simulink model "MX_Gateway"  (Simulink 9.0)
settings: Solver=FixedStepDiscrete  StartTime=0.0  StopTime=100  FixedStep=0.01  RelTol=1e-3
values from neighbouring files (symbols this model uses):
  ACTIVE = Bobcat.Parameter; CreateDefine( ACTIVE                         ,1                          ,'Define' ,'defines.h' )    [defines.m]
  ACTIVE_MODE_ONE = Bobcat.Parameter; CreateDefine( ACTIVE_MODE_ONE                ,19                         ,'Define' ,'defines.h' )    [defines.m]
  AUTHENTICATED = Bobcat.Parameter; CreateDefine( AUTHENTICATED                  ,1                          ,'Define' ,'defines.h' )    [defines.m]
  AUX_EMEA_CONTROLS = Bobcat.Parameter; CreateDefine( AUX_EMEA_CONTROLS  ,1   ,'Define' ,'defines.h' )    [enums.m]
  AUX_FLOW_HIGH = Bobcat.Parameter; CreateDefine( AUX_FLOW_HIGH           ,4  ,'Define' ,'defines.h' )    [enums.m]
  AUX_FLOW_INACTIVE = Bobcat.Parameter; CreateDefine( AUX_FLOW_INACTIVE       ,0  ,'Define' ,'defines.h' )    [enums.m]
  AUX_FLOW_LOW = Bobcat.Parameter; CreateDefine( AUX_FLOW_LOW            ,1  ,'Define' ,'defines.h' )    [enums.m]
  AUX_FLOW_MEDIUM = Bobcat.Parameter; CreateDefine( AUX_FLOW_MEDIUM         ,2  ,'Define' ,'defines.h' )    [enums.m]
  AUX_FLOW_STANDARD = Bobcat.Parameter; CreateDefine( AUX_FLOW_STANDARD       ,3  ,'Define' ,'defines.h' )    [enums.m]
  AUX_NON_FUNCTIONAL = Bobcat.Parameter; CreateDefine( AUX_NON_FUNCTIONAL ,255 ,'Define' ,'defines.h' )    [enums.m]
  BASE_ACTIVE = Bobcat.Parameter; CreateDefine( BASE_ACTIVE                    ,253                        ,'Define' ,'defines.h' )    [defines.m]
  BASE_DETENT = Bobcat.Parameter; CreateDefine( BASE_DETENT                    ,1                          ,'Define' ,'defines.h' )    [defines.m]
  BEEP_NONE = Bobcat.Parameter; CreateDefine( BEEP_NONE                      ,255                        ,'Define' ,'defines.h' )    [defines.m]
  CD_AIR_FILTER_DEBOUNCE_NORMAL = Bobcat.Parameter; CreateAliasCD( CD_AIR_FILTER_DEBOUNCE_NORMAL                        ,uint16(500)                ,'ConstantDataPointer' ,'CONSTANT_DATA' ,'AIR_FILTER_DEBOUNCE_NORMAL')    [cdata.m]    (also in hc12_cdata.m; m4_cdata.m)
  CD_AIR_FILTER_DEBOUNCE_NOT_CONNECTED = Bobcat.Parameter; CreateAliasCD( CD_AIR_FILTER_DEBOUNCE_NOT_CONNECTED                 ,uint16(3000)               ,'ConstantDataPointer' ,'CONSTANT_DATA' ,'AIR_FILTER_DEBOUNCE_NOT_CONNECTED')    [cdata.m]    (also in hc12_cdata.m; m4_cdata.m)
  CD_AIR_FILTER_DEBOUNCE_PLUGGED = Bobcat.Parameter; CreateAliasCD( CD_AIR_FILTER_DEBOUNCE_PLUGGED                       ,uint16(3000)               ,'ConstantDataPointer' ,'CONSTANT_DATA' ,'AIR_FILTER_DEBOUNCE_PLUGGED')    [cdata.m]    (also in hc12_cdata.m; m4_cdata.m)
  CD_AIR_FILTER_FUNCTION = Bobcat.Parameter; CreateAliasCD( CD_AIR_FILTER_FUNCTION                               ,uint8(255)                 ,'ConstantDataPointer' ,'CONSTANT_DATA' ,'AIR_FILTER_FUNCTION')    [cdata.m]    (also in hc12_cdata.m; m4_cdata.m)
  CD_AIR_FILTER_PLUGGED_WEIGHT = Bobcat.Parameter; CreateAliasCD( CD_AIR_FILTER_PLUGGED_WEIGHT                         ,uint8(2)                   ,'ConstantDataPointer' ,'CONSTANT_DATA' ,'AIR_FILTER_PLUGGED_WEIGHT')    [cdata.m]    (also in hc12_cdata.m; m4_cdata.m)
  CD_AIR_FILTER_UNPLUGGED_WEIGHT = Bobcat.Parameter; CreateAliasCD( CD_AIR_FILTER_UNPLUGGED_WEIGHT                       ,uint8(1)                   ,'ConstantDataPointer' ,'CONSTANT_DATA' ,'AIR_FILTER_UNPLUGGED_WEIGHT')    [cdata.m]    (also in hc12_cdata.m; m4_cdata.m)
  CD_AUTOIDLE_ARMBAR_DELAY = Bobcat.Parameter; CreateAliasCD( CD_AUTOIDLE_ARMBAR_DELAY                             ,uint8(2)                   ,'ConstantDataPointer' ,'CONSTANT_DATA' ,'AUTOIDLE_ARMBAR_DELAY')    [cdata.m]    (also in hc12_cdata.m; m4_cdata.m)
  CD_AUTOIDLE_FUNCTION = Bobcat.Parameter; CreateAliasCD( CD_AUTOIDLE_FUNCTION                                 ,uint8(255)                 ,'ConstantDataPointer' ,'CONSTANT_DATA' ,'AUTOIDLE_FUNCTION')    [cdata.m]    (also in hc12_cdata.m; m4_cdata.m)
  CD_AUTOIDLE_IDLE_DELAY = Bobcat.Parameter; CreateAliasCD( CD_AUTOIDLE_IDLE_DELAY                               ,uint8(40)                  ,'ConstantDataPointer' ,'CONSTANT_DATA' ,'AUTOIDLE_IDLE_DELAY')    [cdata.m]    (also in hc12_cdata.m; m4_cdata.m)
  CD_AUTOIDLE_LOW_IDLE_START = Bobcat.Parameter; CreateAliasCD( CD_AUTOIDLE_LOW_IDLE_START                           ,uint8(255)                 ,'ConstantDataPointer' ,'CONSTANT_DATA' ,'AUTOIDLE_LOW_IDLE_START')    [cdata.m]    (also in hc12_cdata.m; m4_cdata.m)
  CD_AUTOIDLE_PRESSURE_OFF = Bobcat.Parameter; CreateAliasCD( CD_AUTOIDLE_PRESSURE_OFF                             ,uint16(300)                ,'ConstantDataPointer' ,'CONSTANT_DATA' ,'AUTOIDLE_PRESSURE_OFF')    [cdata.m]    (also in hc12_cdata.m; m4_cdata.m)
  CD_AUTOIDLE_PRESSURE_ON = Bobcat.Parameter; CreateAliasCD( CD_AUTOIDLE_PRESSURE_ON                              ,uint16(270)                ,'ConstantDataPointer' ,'CONSTANT_DATA' ,'AUTOIDLE_PRESSURE_ON')    [cdata.m]    (also in hc12_cdata.m; m4_cdata.m)
  CD_AUTOSHIFT_FUNCTION = Bobcat.Parameter; CreateAliasCD( CD_AUTOSHIFT_FUNCTION                                ,uint8(255)                 ,'ConstantDataPointer' ,'CONSTANT_DATA' ,'AUTOSHIFT_FUNCTION')    [cdata.m]    (also in hc12_cdata.m; m4_cdata.m)
  CD_AUTOSHIFT_PHIGH1 = Bobcat.Parameter; CreateAliasCD( CD_AUTOSHIFT_PHIGH1                                  ,uint16(205)                ,'ConstantDataPointer' ,'CONSTANT_DATA' ,'AUTOSHIFT_PHIGH1')    [cdata.m]    (also in hc12_cdata.m; m4_cdata.m)
  CD_AUTOSHIFT_PHIGH2 = Bobcat.Parameter; CreateAliasCD( CD_AUTOSHIFT_PHIGH2                                  ,uint16(193)                ,'ConstantDataPointer' ,'CONSTANT_DATA' ,'AUTOSHIFT_PHIGH2')    [cdata.m]    (also in hc12_cdata.m; m4_cdata.m)
  CD_AUTOSHIFT_PHIGH_TEMP1 = Bobcat.Parameter; CreateAliasCD( CD_AUTOSHIFT_PHIGH_TEMP1                             ,int16(70)                  ,'ConstantDataPointer' ,'CONSTANT_DATA' ,'AUTOSHIFT_PHIGH_TEMP1')    [cdata.m]    (also in hc12_cdata.m; m4_cdata.m)
  CD_AUTOSHIFT_PHIGH_TEMP2 = Bobcat.Parameter; CreateAliasCD( CD_AUTOSHIFT_PHIGH_TEMP2                             ,int16(160)                 ,'ConstantDataPointer' ,'CONSTANT_DATA' ,'AUTOSHIFT_PHIGH_TEMP2')    [cdata.m]    (also in hc12_cdata.m; m4_cdata.m)
  CD_AUTOSHIFT_PLOW1 = Bobcat.Parameter; CreateAliasCD( CD_AUTOSHIFT_PLOW1                                   ,uint16(130)                ,'ConstantDataPointer' ,'CONSTANT_DATA' ,'AUTOSHIFT_PLOW1')    [cdata.m]    (also in hc12_cdata.m; m4_cdata.m)
  CD_AUTOSHIFT_PLOW2 = Bobcat.Parameter; CreateAliasCD( CD_AUTOSHIFT_PLOW2                                   ,uint16(100)                ,'ConstantDataPointer' ,'CONSTANT_DATA' ,'AUTOSHIFT_PLOW2')    [cdata.m]    (also in hc12_cdata.m; m4_cdata.m)
  CD_AUTOSHIFT_PLOW_TEMP1 = Bobcat.Parameter; CreateAliasCD( CD_AUTOSHIFT_PLOW_TEMP1                              ,int16(20)                  ,'ConstantDataPointer' ,'CONSTANT_DATA' ,'AUTOSHIFT_PLOW_TEMP1')    [cdata.m]    (also in hc12_cdata.m; m4_cdata.m)
  CD_AUTOSHIFT_PLOW_TEMP2 = Bobcat.Parameter; CreateAliasCD( CD_AUTOSHIFT_PLOW_TEMP2                              ,int16(80)                  ,'ConstantDataPointer' ,'CONSTANT_DATA' ,'AUTOSHIFT_PLOW_TEMP2')    [cdata.m]    (also in hc12_cdata.m; m4_cdata.m)
  CD_AUTOSHIFT_TRAVEL_DELAY = Bobcat.Parameter; CreateAliasCD( CD_AUTOSHIFT_TRAVEL_DELAY                            ,uint8(32)                  ,'ConstantDataPointer' ,'CONSTANT_DATA' ,'AUTOSHIFT_TRAVEL_DELAY')    [cdata.m]    (also in hc12_cdata.m; m4_cdata.m)
  CD_AUX2_CRACKPOINT_CURRENT = Bobcat.Parameter; CreateAliasCD( CD_AUX2_CRACKPOINT_CURRENT                           ,fi(0,0,16,12,'hex','0c28') ,'ConstantDataPointer' ,'CONSTANT_DATA' ,'AUX2_CRACKPOINT_CURRENT')    [cdata.m]    (also in hc12_cdata.m; m4_cdata.m)
  CD_AUX2_FLOW_LOW_FUNCTION = Bobcat.Parameter; CreateAliasCD( CD_AUX2_FLOW_LOW_FUNCTION                            ,uint8(255)                 ,'ConstantDataPointer' ,'CONSTANT_DATA' ,'AUX2_FLOW_LOW_FUNCTION')    [cdata.m]    (also in hc12_cdata.m; m4_cdata.m)
  CD_AUX2_FLOW_MED_FUNCTION = Bobcat.Parameter; CreateAliasCD( CD_AUX2_FLOW_MED_FUNCTION                            ,uint8(255)                 ,'ConstantDataPointer' ,'CONSTANT_DATA' ,'AUX2_FLOW_MED_FUNCTION')    [cdata.m]    (also in hc12_cdata.m; m4_cdata.m)
  CD_AUX2_HI_FLOW_PERCENT = Bobcat.Parameter; CreateAliasCD( CD_AUX2_HI_FLOW_PERCENT                              ,fi(0,0,16,16,'hex','ffff') ,'ConstantDataPointer' ,'CONSTANT_DATA' ,'AUX2_HI_FLOW_PERCENT')    [cdata.m]    (also in hc12_cdata.m; m4_cdata.m)
  CD_AUX2_LOW_FLOW_PERCENT = Bobcat.Parameter; CreateAliasCD( CD_AUX2_LOW_FLOW_PERCENT                             ,fi(0,0,16,16,'hex','7333') ,'ConstantDataPointer' ,'CONSTANT_DATA' ,'AUX2_LOW_FLOW_PERCENT')    [cdata.m]    (also in hc12_cdata.m; m4_cdata.m)
  CD_AUX2_MAX_FLOW_CURRENT = Bobcat.Parameter; CreateAliasCD( CD_AUX2_MAX_FLOW_CURRENT                             ,fi(0,0,16,12,'hex','14cc') ,'ConstantDataPointer' ,'CONSTANT_DATA' ,'AUX2_MAX_FLOW_CURRENT')    [cdata.m]    (also in hc12_cdata.m; m4_cdata.m)
  CD_AUX2_MED_FLOW_PERCENT = Bobcat.Parameter; CreateAliasCD( CD_AUX2_MED_FLOW_PERCENT                             ,fi(0,0,16,16,'hex','9999') ,'ConstantDataPointer' ,'CONSTANT_DATA' ,'AUX2_MED_FLOW_PERCENT')    [cdata.m]    (also in hc12_cdata.m; m4_cdata.m)
  CD_AUX_CRACKPOINT_CURRENT = Bobcat.Parameter; CreateAliasCD( CD_AUX_CRACKPOINT_CURRENT                            ,fi(0,0,16,12,'hex','0000') ,'ConstantDataPointer' ,'CONSTANT_DATA' ,'AUX_CRACKPOINT_CURRENT')    [cdata.m]    (also in hc12_cdata.m; m4_cdata.m)
  CD_AUX_DETENT_FLASH_TIME = Bobcat.Parameter; CreateAliasCD( CD_AUX_DETENT_FLASH_TIME                             ,uint16(72)                 ,'ConstantDataPointer' ,'CONSTANT_DATA' ,'AUX_DETENT_FLASH_TIME')    [cdata.m]    (also in hc12_cdata.m; m4_cdata.m)
  CD_AUX_DETENT_HOLD_TIME = Bobcat.Parameter; CreateAliasCD( CD_AUX_DETENT_HOLD_TIME                              ,uint16(100)                ,'ConstantDataPointer' ,'CONSTANT_DATA' ,'AUX_DETENT_HOLD_TIME')    [cdata.m]    (also in hc12_cdata.m; m4_cdata.m)
  CD_AUX_FULL_ON_AUX1 = Bobcat.Parameter; CreateAliasCD( CD_AUX_FULL_ON_AUX1                                  ,fi(0,0,16,12,'hex','10cd') ,'ConstantDataPointer' ,'CONSTANT_DATA' ,'AUX_FULL_ON_AUX1')    [cdata.m]    (also in hc12_cdata.m; m4_cdata.m)
  CD_AUX_FULL_ON_AUX2 = Bobcat.Parameter; CreateAliasCD( CD_AUX_FULL_ON_AUX2                                  ,fi(0,0,16,12,'hex','139d') ,'ConstantDataPointer' ,'CONSTANT_DATA' ,'AUX_FULL_ON_AUX2')    [cdata.m]    (also in hc12_cdata.m; m4_cdata.m)
  CD_AUX_FULL_ON_AUX3 = Bobcat.Parameter; CreateAliasCD( CD_AUX_FULL_ON_AUX3                                  ,fi(0,0,16,12,'hex','17d7') ,'ConstantDataPointer' ,'CONSTANT_DATA' ,'AUX_FULL_ON_AUX3')    [cdata.m]    (also in hc12_cdata.m; m4_cdata.m)
  CD_AUX_FUNCTION = Bobcat.Parameter; CreateAliasCD( CD_AUX_FUNCTION                                      ,uint8(0)                   ,'ConstantDataPointer' ,'CONSTANT_DATA' ,'AUX_FUNCTION')    [cdata.m]    (also in hc12_cdata.m; m4_cdata.m)
  CD_AUX_RATE_LIMIT_CRACKPOINT = Bobcat.Parameter; CreateAliasCD( CD_AUX_RATE_LIMIT_CRACKPOINT                         ,fi(0,0,16,12,'hex','0800') ,'ConstantDataPointer' ,'CONSTANT_DATA' ,'AUX_RATE_LIMIT_CRACKPOINT')    [cdata.m]    (also in hc12_cdata.m; m4_cdata.m)
  CD_AUX_RATE_LIMIT_CRACKPOINT_RATE = Bobcat.Parameter; CreateAliasCD( CD_AUX_RATE_LIMIT_CRACKPOINT_RATE                    ,fi(0,1,32,12,'int',102400) ,'ConstantDataPointer' ,'CONSTANT_DATA' ,'AUX_RATE_LIMIT_CRACKPOINT_RATE')    [cdata.m]    (also in hc12_cdata.m; m4_cdata.m)
  CD_AUX_RATE_LIMIT_FUNCTION = Bobcat.Parameter; CreateAliasCD( CD_AUX_RATE_LIMIT_FUNCTION                           ,uint8(255)                 ,'ConstantDataPointer' ,'CONSTANT_DATA' ,'AUX_RATE_LIMIT_FUNCTION')    [cdata.m]    (also in hc12_cdata.m; m4_cdata.m)
  CD_AUX_RATE_LIMIT_MAX_ENG_SPD = Bobcat.Parameter; CreateAliasCD( CD_AUX_RATE_LIMIT_MAX_ENG_SPD                        ,uint16(1600)               ,'ConstantDataPointer' ,'CONSTANT_DATA' ,'AUX_RATE_LIMIT_MAX_ENG_SPD')    [cdata.m]    (also in hc12_cdata.m; m4_cdata.m)
  CD_AUX_RATE_LIMIT_MIN_ENG_SPD = Bobcat.Parameter; CreateAliasCD( CD_AUX_RATE_LIMIT_MIN_ENG_SPD                        ,uint16(100)                ,'ConstantDataPointer' ,'CONSTANT_DATA' ,'AUX_RATE_LIMIT_MIN_ENG_SPD')    [cdata.m]    (also in hc12_cdata.m; m4_cdata.m)
  CD_AUX_RATE_LIMIT_NORMAL_RATE = Bobcat.Parameter; CreateAliasCD( CD_AUX_RATE_LIMIT_NORMAL_RATE                        ,fi(0,1,32,12,'int',2048)   ,'ConstantDataPointer' ,'CONSTANT_DATA' ,'AUX_RATE_LIMIT_NORMAL_RATE')    [cdata.m]    (also in hc12_cdata.m; m4_cdata.m)
  CD_AUXPOT_CAL_DEADBAND_HIGH = Bobcat.Parameter; CreateAliasCD( CD_AUXPOT_CAL_DEADBAND_HIGH                          ,uint16(574)                ,'ConstantDataPointer' ,'CONSTANT_DATA' ,'AUXPOT_CAL_DEADBAND_HIGH')    [cdata.m]    (also in hc12_cdata.m; m4_cdata.m)
  CD_AUXPOT_CAL_DEADBAND_LOW = Bobcat.Parameter; CreateAliasCD( CD_AUXPOT_CAL_DEADBAND_LOW                           ,uint16(424)                ,'ConstantDataPointer' ,'CONSTANT_DATA' ,'AUXPOT_CAL_DEADBAND_LOW')    [cdata.m]    (also in hc12_cdata.m; m4_cdata.m)
  CD_AUXPOT_CENTER_RATIO = Bobcat.Parameter; CreateAliasCD( CD_AUXPOT_CENTER_RATIO                               ,uint16(500)                ,'ConstantDataPointer' ,'CONSTANT_DATA' ,'AUXPOT_CENTER_RATIO')    [cdata.m]    (also in hc12_cdata.m; m4_cdata.m)
  CD_AUXPOT_DEADBAND = Bobcat.Parameter; CreateAliasCD( CD_AUXPOT_DEADBAND                                   ,uint8(38)                  ,'ConstantDataPointer' ,'CONSTANT_DATA' ,'AUXPOT_DEADBAND')    [cdata.m]    (also in hc12_cdata.m; m4_cdata.m)
  CD_AUXPOT_FUNCTION = Bobcat.Parameter; CreateAliasCD( CD_AUXPOT_FUNCTION                                   ,uint8(0)                   ,'ConstantDataPointer' ,'CONSTANT_DATA' ,'AUXPOT_FUNCTION')    [cdata.m]    (also in hc12_cdata.m; m4_cdata.m)
  CD_AUXPOT_RATIO_GAIN = Bobcat.Parameter; CreateAliasCD( CD_AUXPOT_RATIO_GAIN                                 ,uint16(1000)               ,'ConstantDataPointer' ,'CONSTANT_DATA' ,'AUXPOT_RATIO_GAIN')    [cdata.m]    (also in hc12_cdata.m; m4_cdata.m)
  CD_CAN_THROTTLE_FUNCTION = Bobcat.Parameter; CreateAliasCD( CD_CAN_THROTTLE_FUNCTION                             ,uint8(255)                 ,'ConstantDataPointer' ,'CONSTANT_DATA' ,'CAN_THROTTLE_FUNCTION')    [cdata.m]    (also in hc12_cdata.m; m4_cdata.m)
  CD_CAN_THROTTLE_REVERSE = Bobcat.Parameter; CreateAliasCD( CD_CAN_THROTTLE_REVERSE                              ,uint8(255)                 ,'ConstantDataPointer' ,'CONSTANT_DATA' ,'CAN_THROTTLE_REVERSE')    [cdata.m]    (also in hc12_cdata.m; m4_cdata.m)
  CD_CONSOLE_DEBOUNCE_ABSENT = Bobcat.Parameter; CreateAliasCD( CD_CONSOLE_DEBOUNCE_ABSENT                           ,uint16(12)                 ,'ConstantDataPointer' ,'CONSTANT_DATA' ,'CONSOLE_DEBOUNCE_ABSENT')    [cdata.m]    (also in hc12_cdata.m; m4_cdata.m)
  CD_CONSOLE_DEBOUNCE_OORH = Bobcat.Parameter; CreateAliasCD( CD_CONSOLE_DEBOUNCE_OORH                             ,uint16(12)                 ,'ConstantDataPointer' ,'CONSTANT_DATA' ,'CONSOLE_DEBOUNCE_OORH')    [cdata.m]    (also in hc12_cdata.m; m4_cdata.m)
  CD_CONSOLE_DEBOUNCE_OORL = Bobcat.Parameter; CreateAliasCD( CD_CONSOLE_DEBOUNCE_OORL                             ,uint16(12)                 ,'ConstantDataPointer' ,'CONSTANT_DATA' ,'CONSOLE_DEBOUNCE_OORL')    [cdata.m]    (also in hc12_cdata.m; m4_cdata.m)
  CD_CONSOLE_DEBOUNCE_PRESENT = Bobcat.Parameter; CreateAliasCD( CD_CONSOLE_DEBOUNCE_PRESENT                          ,uint16(12)                 ,'ConstantDataPointer' ,'CONSTANT_DATA' ,'CONSOLE_DEBOUNCE_PRESENT')    [cdata.m]    (also in hc12_cdata.m; m4_cdata.m)
  CD_CONSOLE_FUNCTION = Bobcat.Parameter; CreateAliasCD( CD_CONSOLE_FUNCTION                                  ,uint8(0)                   ,'ConstantDataPointer' ,'CONSTANT_DATA' ,'CONSOLE_FUNCTION')    [cdata.m]    (also in hc12_cdata.m; m4_cdata.m)
  CD_CONSOLE_OORH = Bobcat.Parameter; CreateAliasCD( CD_CONSOLE_OORH                                      ,uint16(740)                ,'ConstantDataPointer' ,'CONSTANT_DATA' ,'CONSOLE_OORH')    [cdata.m]    (also in hc12_cdata.m; m4_cdata.m)
  CD_CONSOLE_OORL = Bobcat.Parameter; CreateAliasCD( CD_CONSOLE_OORL                                      ,uint16(10)                 ,'ConstantDataPointer' ,'CONSTANT_DATA' ,'CONSOLE_OORL')    [cdata.m]    (also in hc12_cdata.m; m4_cdata.m)
  CD_CONSOLE_PRESENT_HIGH = Bobcat.Parameter; CreateAliasCD( CD_CONSOLE_PRESENT_HIGH                              ,uint16(515)                ,'ConstantDataPointer' ,'CONSTANT_DATA' ,'CONSOLE_PRESENT_HIGH')    [cdata.m]    (also in hc12_cdata.m; m4_cdata.m)
  CD_CONSOLE_PRESENT_LOW = Bobcat.Parameter; CreateAliasCD( CD_CONSOLE_PRESENT_LOW                               ,uint16(375)                ,'ConstantDataPointer' ,'CONSTANT_DATA' ,'CONSOLE_PRESENT_LOW')    [cdata.m]    (also in hc12_cdata.m; m4_cdata.m)
  CD_DELUXE_GEN5_5_FUNCTION = Bobcat.Parameter; CreateAliasCD( CD_DELUXE_GEN5_5_FUNCTION                            ,uint8(255)                 ,'ConstantDataPointer' ,'CONSTANT_DATA' ,'DELUXE_GEN5_5_FUNCTION')    [cdata.m]    (also in hc12_cdata.m; m4_cdata.m)
  CD_DELUXE_GEN5_FUNCTION = Bobcat.Parameter; CreateAliasCD( CD_DELUXE_GEN5_FUNCTION                              ,uint8(255)                 ,'ConstantDataPointer' ,'CONSTANT_DATA' ,'DELUXE_GEN5_FUNCTION')    [cdata.m]    (also in hc12_cdata.m; m4_cdata.m)
  CD_DERATE_ENGINE_SPEED_DEBOUNCE_HIGH = Bobcat.Parameter; CreateAliasCD( CD_DERATE_ENGINE_SPEED_DEBOUNCE_HIGH                 ,uint16(200)                ,'ConstantDataPointer' ,'CONSTANT_DATA' ,'DERATE_ENGINE_SPEED_DEBOUNCE_HIGH')    [cdata.m]    (also in hc12_cdata.m; m4_cdata.m)
  CD_DERATE_ENGINE_SPEED_DEBOUNCE_SHUTDOWN = Bobcat.Parameter; CreateAliasCD( CD_DERATE_ENGINE_SPEED_DEBOUNCE_SHUTDOWN             ,uint16(1000)               ,'ConstantDataPointer' ,'CONSTANT_DATA' ,'DERATE_ENGINE_SPEED_DEBOUNCE_SHUTDOWN')    [cdata.m]    (also in hc12_cdata.m; m4_cdata.m)
  CD_DERATE_ENGINE_SPEED_HIGH_OFFSET = Bobcat.Parameter; CreateAliasCD( CD_DERATE_ENGINE_SPEED_HIGH_OFFSET                   ,uint16(200)                ,'ConstantDataPointer' ,'CONSTANT_DATA' ,'DERATE_ENGINE_SPEED_HIGH_OFFSET')    [cdata.m]    (also in hc12_cdata.m; m4_cdata.m)
  CD_DERATE_METHOD = Bobcat.Parameter; CreateAliasCD( CD_DERATE_METHOD                                     ,uint8(0)                   ,'ConstantDataPointer' ,'CONSTANT_DATA' ,'DERATE_METHOD')    [cdata.m]    (also in hc12_cdata.m; m4_cdata.m)
  CD_DUMP_VALVE_FUNCTION = Bobcat.Parameter; CreateAliasCD( CD_DUMP_VALVE_FUNCTION                               ,uint8(255)                 ,'ConstantDataPointer' ,'CONSTANT_DATA' ,'DUMP_VALVE_FUNCTION')    [cdata.m]    (also in hc12_cdata.m; m4_cdata.m)
  CD_DUMP_VALVE_ON_CURRENT = Bobcat.Parameter; CreateAliasCD( CD_DUMP_VALVE_ON_CURRENT                             ,fi(0,0,16,12,'hex','ffff') ,'ConstantDataPointer' ,'CONSTANT_DATA' ,'DUMP_VALVE_ON_CURRENT')    [cdata.m]    (also in hc12_cdata.m; m4_cdata.m)
  CD_ECO_MODE_THROTTLE_REDUCTION = Bobcat.Parameter; CreateAliasCD( CD_ECO_MODE_THROTTLE_REDUCTION                       ,uint16(850)                ,'ConstantDataPointer' ,'CONSTANT_DATA' ,'ECO_MODE_THROTTLE_REDUCTION')    [cdata.m]    (also in hc12_cdata.m; m4_cdata.m)
  CD_ECT_FILTER_GAIN = Bobcat.Parameter; CreateAliasCD( CD_ECT_FILTER_GAIN                                   ,uint8(6)                   ,'ConstantDataPointer' ,'CONSTANT_DATA' ,'ECT_FILTER_GAIN')    [cdata.m]    (also in hc12_cdata.m; m4_cdata.m)
  CD_ECU_FUNCTION = Bobcat.Parameter; CreateAliasCD( CD_ECU_FUNCTION                                      ,uint8(255)                 ,'ConstantDataPointer' ,'CONSTANT_DATA' ,'ECU_FUNCTION')    [cdata.m]    (also in hc12_cdata.m; m4_cdata.m)
  CD_ECU_HOLD_DEBOUNCE_ERROR_ON = Bobcat.Parameter; CreateAliasCD( CD_ECU_HOLD_DEBOUNCE_ERROR_ON                        ,uint16(20)                 ,'ConstantDataPointer' ,'CONSTANT_DATA' ,'ECU_HOLD_DEBOUNCE_ERROR_ON')    [cdata.m]    (also in hc12_cdata.m; m4_cdata.m)
  CD_EMEA_CONTROLS_HOLD_TIME = Bobcat.Parameter; CreateAliasCD( CD_EMEA_CONTROLS_HOLD_TIME                           ,uint16(30)                 ,'ConstantDataPointer' ,'CONSTANT_DATA' ,'EMEA_CONTROLS_HOLD_TIME')    [cdata.m]    (also in hc12_cdata.m; m4_cdata.m)
  CD_EMEA_CONTROLS_OPTION_A = Bobcat.Parameter; CreateAliasCD( CD_EMEA_CONTROLS_OPTION_A                            ,uint8(255)                 ,'ConstantDataPointer' ,'CONSTANT_DATA' ,'EMEA_CONTROLS_OPTION_A')    [cdata.m]    (also in hc12_cdata.m; m4_cdata.m)
  CD_EMEA_CONTROLS_OPTION_C = Bobcat.Parameter; CreateAliasCD( CD_EMEA_CONTROLS_OPTION_C                            ,uint8(255)                 ,'ConstantDataPointer' ,'CONSTANT_DATA' ,'EMEA_CONTROLS_OPTION_C')    [cdata.m]    (also in hc12_cdata.m; m4_cdata.m)
  CD_EMEA_CONTROLS_REVERSE = Bobcat.Parameter; CreateAliasCD( CD_EMEA_CONTROLS_REVERSE                             ,uint8(255)                 ,'ConstantDataPointer' ,'CONSTANT_DATA' ,'EMEA_CONTROLS_REVERSE')    [cdata.m]    (also in hc12_cdata.m; m4_cdata.m)
  CD_EMEA_ELECTRIC_VERSION = Bobcat.Parameter; CreateAliasCD( CD_EMEA_ELECTRIC_VERSION                             ,uint8(0)                   ,'ConstantDataPointer' ,'CONSTANT_DATA' ,'EMEA_ELECTRIC_VERSION')    [cdata.m]    (also in hc12_cdata.m; m4_cdata.m)
  CD_EMEA_POT_CAL_DEADBAND_HIGH = Bobcat.Parameter; CreateAliasCD( CD_EMEA_POT_CAL_DEADBAND_HIGH                        ,uint16(574)                ,'ConstantDataPointer' ,'CONSTANT_DATA' ,'EMEA_POT_CAL_DEADBAND_HIGH')    [cdata.m]    (also in hc12_cdata.m; m4_cdata.m)
  CD_EMEA_POT_CAL_DEADBAND_LOW = Bobcat.Parameter; CreateAliasCD( CD_EMEA_POT_CAL_DEADBAND_LOW                         ,uint16(424)                ,'ConstantDataPointer' ,'CONSTANT_DATA' ,'EMEA_POT_CAL_DEADBAND_LOW')    [cdata.m]    (also in hc12_cdata.m; m4_cdata.m)
  CD_ENGINE_RPM_FACTOR = Bobcat.Parameter; CreateAliasCD( CD_ENGINE_RPM_FACTOR                                 ,uint8(35)                  ,'ConstantDataPointer' ,'CONSTANT_DATA' ,'ENGINE_RPM_FACTOR')    [cdata.m]    (also in hc12_cdata.m; m4_cdata.m)
  CD_ENGINE_SPEED_FILTER_FAST_VALUE = Bobcat.Parameter; CreateAliasCD( CD_ENGINE_SPEED_FILTER_FAST_VALUE                    ,uint8(1)                   ,'ConstantDataPointer' ,'CONSTANT_DATA' ,'ENGINE_SPEED_FILTER_FAST_VALUE')    [cdata.m]    (also in hc12_cdata.m; m4_cdata.m)
  CD_ENGINE_SPEED_FILTER_SLOW_VALUE = Bobcat.Parameter; CreateAliasCD( CD_ENGINE_SPEED_FILTER_SLOW_VALUE                    ,uint8(4)                   ,'ConstantDataPointer' ,'CONSTANT_DATA' ,'ENGINE_SPEED_FILTER_SLOW_VALUE')    [cdata.m]    (also in hc12_cdata.m; m4_cdata.m)
  CD_ENGINE_SPEED_RUNNING = Bobcat.Parameter; CreateAliasCD( CD_ENGINE_SPEED_RUNNING                              ,uint16(800)                ,'ConstantDataPointer' ,'CONSTANT_DATA' ,'ENGINE_SPEED_RUNNING')    [cdata.m]    (also in hc12_cdata.m; m4_cdata.m)
  CD_ENGINE_START_DELAY_DEBOUNCE_RPM_NO_SIGNAL = Bobcat.Parameter; CreateAliasCD( CD_ENGINE_START_DELAY_DEBOUNCE_RPM_NO_SIGNAL         ,uint16(0)                  ,'ConstantDataPointer' ,'CONSTANT_DATA' ,'ENGINE_START_DELAY_DEBOUNCE_RPM_NO_SIGNAL')    [cdata.m]    (also in hc12_cdata.m; m4_cdata.m)
  CD_ENGINE_START_DELAY_OFF_TIME = Bobcat.Parameter; CreateAliasCD( CD_ENGINE_START_DELAY_OFF_TIME                       ,uint16(0)                  ,'ConstantDataPointer' ,'CONSTANT_DATA' ,'ENGINE_START_DELAY_OFF_TIME')    [cdata.m]    (also in hc12_cdata.m; m4_cdata.m)
  CD_ENGINE_START_DELAY_RPM_NO_SIGNAL = Bobcat.Parameter; CreateAliasCD( CD_ENGINE_START_DELAY_RPM_NO_SIGNAL                  ,uint16(0)                  ,'ConstantDataPointer' ,'CONSTANT_DATA' ,'ENGINE_START_DELAY_RPM_NO_SIGNAL')    [cdata.m]    (also in hc12_cdata.m; m4_cdata.m)
  CD_EOP_DEBOUNCE_EXTREMELY_LOW = Bobcat.Parameter; CreateAliasCD( CD_EOP_DEBOUNCE_EXTREMELY_LOW                        ,uint16(50)                 ,'ConstantDataPointer' ,'CONSTANT_DATA' ,'EOP_DEBOUNCE_EXTREMELY_LOW')    [cdata.m]    (also in hc12_cdata.m; m4_cdata.m)
  CD_EOP_DEBOUNCE_NORMAL = Bobcat.Parameter; CreateAliasCD( CD_EOP_DEBOUNCE_NORMAL                               ,uint16(50)                 ,'ConstantDataPointer' ,'CONSTANT_DATA' ,'EOP_DEBOUNCE_NORMAL')    [cdata.m]    (also in hc12_cdata.m; m4_cdata.m)
  CD_EOP_DEBOUNCE_SHUTDOWN = Bobcat.Parameter; CreateAliasCD( CD_EOP_DEBOUNCE_SHUTDOWN                             ,uint16(100)                ,'ConstantDataPointer' ,'CONSTANT_DATA' ,'EOP_DEBOUNCE_SHUTDOWN')    [cdata.m]    (also in hc12_cdata.m; m4_cdata.m)
  CD_EOP_FILTER_VALUE = Bobcat.Parameter; CreateAliasCD( CD_EOP_FILTER_VALUE                                  ,uint8(3)                   ,'ConstantDataPointer' ,'CONSTANT_DATA' ,'EOP_FILTER_VALUE')    [cdata.m]    (also in hc12_cdata.m; m4_cdata.m)
  CD_EOP_FUNCTION = Bobcat.Parameter; CreateAliasCD( CD_EOP_FUNCTION                                      ,uint8(1)                   ,'ConstantDataPointer' ,'CONSTANT_DATA' ,'EOP_FUNCTION')    [cdata.m]    (also in hc12_cdata.m; m4_cdata.m)
  CD_EOP_SWITCH_GAUGE_VALUE = Bobcat.Parameter; CreateAliasCD( CD_EOP_SWITCH_GAUGE_VALUE                            ,uint16(125)                ,'ConstantDataPointer' ,'CONSTANT_DATA' ,'EOP_SWITCH_GAUGE_VALUE')    [cdata.m]    (also in hc12_cdata.m; m4_cdata.m)
  CD_EOP_SWITCH_LOW = Bobcat.Parameter; CreateAliasCD( CD_EOP_SWITCH_LOW                                    ,uint16(50)                 ,'ConstantDataPointer' ,'CONSTANT_DATA' ,'EOP_SWITCH_LOW')    [cdata.m]    (also in hc12_cdata.m; m4_cdata.m)
  CD_EOP_SWITCH_PRESSURE_VALUE = Bobcat.Parameter; CreateAliasCD( CD_EOP_SWITCH_PRESSURE_VALUE                         ,uint16(40)                 ,'ConstantDataPointer' ,'CONSTANT_DATA' ,'EOP_SWITCH_PRESSURE_VALUE')    [cdata.m]    (also in hc12_cdata.m; m4_cdata.m)
  CD_EOT_DEBOUNCE_EXTREMELY_LOW = Bobcat.Parameter; CreateAliasCD( CD_EOT_DEBOUNCE_EXTREMELY_LOW                        ,uint16(2)                  ,'ConstantDataPointer' ,'CONSTANT_DATA' ,'EOT_DEBOUNCE_EXTREMELY_LOW')    [cdata.m]    (also in hc12_cdata.m; m4_cdata.m)
  CD_EOT_DEBOUNCE_LOW = Bobcat.Parameter; CreateAliasCD( CD_EOT_DEBOUNCE_LOW                                  ,uint16(2)                  ,'ConstantDataPointer' ,'CONSTANT_DATA' ,'EOT_DEBOUNCE_LOW')    [cdata.m]    (also in hc12_cdata.m; m4_cdata.m)
  CD_EOT_DEBOUNCE_NORMAL = Bobcat.Parameter; CreateAliasCD( CD_EOT_DEBOUNCE_NORMAL                               ,uint16(2)                  ,'ConstantDataPointer' ,'CONSTANT_DATA' ,'EOT_DEBOUNCE_NORMAL')    [cdata.m]    (also in hc12_cdata.m; m4_cdata.m)
  CD_EOT_EXTREMELY_LOW = Bobcat.Parameter; CreateAliasCD( CD_EOT_EXTREMELY_LOW                                 ,uint16(81)                 ,'ConstantDataPointer' ,'CONSTANT_DATA' ,'EOT_EXTREMELY_LOW')    [cdata.m]    (also in hc12_cdata.m; m4_cdata.m)
  CD_EOT_FUNCTION = Bobcat.Parameter; CreateAliasCD( CD_EOT_FUNCTION                                      ,uint8(255)                 ,'ConstantDataPointer' ,'CONSTANT_DATA' ,'EOT_FUNCTION')    [cdata.m]    (also in hc12_cdata.m; m4_cdata.m)
  CD_EOT_LOW = Bobcat.Parameter; CreateAliasCD( CD_EOT_LOW                                           ,uint16(90)                 ,'ConstantDataPointer' ,'CONSTANT_DATA' ,'EOT_LOW')    [cdata.m]    (also in hc12_cdata.m; m4_cdata.m)
  CD_EOT_TEMPERATURE_HYSTERESIS = Bobcat.Parameter; CreateAliasCD( CD_EOT_TEMPERATURE_HYSTERESIS                        ,uint16(2)                  ,'ConstantDataPointer' ,'CONSTANT_DATA' ,'EOT_TEMPERATURE_HYSTERESIS')    [cdata.m]    (also in hc12_cdata.m; m4_cdata.m)
  CD_FAST_TRAVEL_FUNCTION = Bobcat.Parameter; CreateAliasCD( CD_FAST_TRAVEL_FUNCTION                              ,uint8(255)                 ,'ConstantDataPointer' ,'CONSTANT_DATA' ,'FAST_TRAVEL_FUNCTION')    [cdata.m]    (also in hc12_cdata.m; m4_cdata.m)
  CD_FAST_TRAVEL_THROTTLE_DELAY = Bobcat.Parameter; CreateAliasCD( CD_FAST_TRAVEL_THROTTLE_DELAY                        ,uint16(10)                 ,'ConstantDataPointer' ,'CONSTANT_DATA' ,'FAST_TRAVEL_THROTTLE_DELAY')    [cdata.m]    (also in hc12_cdata.m; m4_cdata.m)
  CD_FAST_TRAVEL_THROTTLE_LIMIT = Bobcat.Parameter; CreateAliasCD( CD_FAST_TRAVEL_THROTTLE_LIMIT                        ,uint16(1000)               ,'ConstantDataPointer' ,'CONSTANT_DATA' ,'FAST_TRAVEL_THROTTLE_LIMIT')    [cdata.m]    (also in hc12_cdata.m; m4_cdata.m)
  CD_FLYWHEEL_TEETH = Bobcat.Parameter; CreateAliasCD( CD_FLYWHEEL_TEETH                                    ,uint8(83)                  ,'ConstantDataPointer' ,'CONSTANT_DATA' ,'FLYWHEEL_TEETH')    [cdata.m]    (also in hc12_cdata.m; m4_cdata.m)
  CD_FUEL_LEVEL_FILTER_FAST_VALUE = Bobcat.Parameter; CreateAliasCD( CD_FUEL_LEVEL_FILTER_FAST_VALUE                      ,uint8(5)                   ,'ConstantDataPointer' ,'CONSTANT_DATA' ,'FUEL_LEVEL_FILTER_FAST_VALUE')    [cdata.m]    (also in hc12_cdata.m; m4_cdata.m)
  CD_FUEL_LEVEL_FILTER_SLOW_VALUE = Bobcat.Parameter; CreateAliasCD( CD_FUEL_LEVEL_FILTER_SLOW_VALUE                      ,uint8(10)                  ,'ConstantDataPointer' ,'CONSTANT_DATA' ,'FUEL_LEVEL_FILTER_SLOW_VALUE')    [cdata.m]    (also in hc12_cdata.m; m4_cdata.m)
  CD_FUEL_PRIMARY_FEEDBACK_FUNCTION = Bobcat.Parameter; CreateAliasCD( CD_FUEL_PRIMARY_FEEDBACK_FUNCTION                    ,uint8(0)                   ,'ConstantDataPointer' ,'CONSTANT_DATA' ,'FUEL_PRIMARY_FEEDBACK_FUNCTION')    [cdata.m]    (also in hc12_cdata.m; m4_cdata.m)
  ... (+246 more)
undefined symbols (not defined in the file or in its folder): sigbuilder_block
undefined symbols (not defined in the file): CD_FUEL_RESOLUTION_TIMER
libraries: simulink x186, MX_Library x107
(part 38 of 48)

== stateflow chart MX_Gtwy_Control/Outputs/Diverter/Diverter/Diverter ==
  state NON_FUNCTIONAL
    en:
    cmd_Diverter = INACTIVE;
  state EMEA_CONTROLS
    state INACTIVE
      en:
      cmd_Diverter = INACTIVE;
    state ACTIVE
      en:
      cmd_Diverter = ACTIVE;
    (default) -> INACTIVE
    INACTIVE -> ACTIVE : [mode_LeftControl == MODE_LEFT_AUX2...
        | && state_Diverter == INACTIVE]
    ACTIVE -> INACTIVE : [mode_LeftControl != MODE_LEFT_AUX2...
        | || state_Diverter != ACTIVE]
  state SECOND_AUX_NA
    state InactiveCheck
      en:
      cmd_Diverter = INACTIVE;
    state Inactive
      en:
      cmd_Diverter = INACTIVE;
    state DebounceActive
    state Active
      en:
      cmd_Diverter = ACTIVE;
    state DebounceInactive
    (default) -> InactiveCheck
    InactiveCheck -> Inactive : [din_SecondAuxSw == INACTIVE]
    Inactive -> DebounceActive [order 2] : [din_SecondAuxSw == ACTIVE...
        | && state_Diverter == INACTIVE...
        | && switch_hold == INACTIVE]
    Inactive -> Inactive [order 1] : [din_SecondAuxSw == INACTIVE]
        | {
        |   switch_hold = INACTIVE;
        | }
    DebounceActive -> Inactive [order 1] : [din_SecondAuxSw == INACTIVE]
    DebounceInactive -> Inactive [order 2] : [after(CD_SECOND_AUX_SWITCH_DEBOUNCE,tick)]
        | {
        | state_Buzzer = SINGLE_SHORT_BEEP;
        | switch_hold = ACTIVE;
        | }
    Active -> Inactive [order 3] : [state_Diverter != ACTIVE...
        | && state_Diverter != INACTIVE]
    DebounceActive -> Active [order 2] : [after(CD_SECOND_AUX_SWITCH_DEBOUNCE,tick)]
        | {
        |   state_Buzzer = SINGLE_SHORT_BEEP;
        |   switch_hold = ACTIVE;
        | }
    Active -> DebounceInactive [order 2] : [din_SecondAuxSw == ACTIVE...
        | && switch_hold == INACTIVE]
    DebounceInactive -> Active [order 1] : [din_SecondAuxSw == INACTIVE]
    Active -> Active [order 1] : [din_SecondAuxSw == INACTIVE]
        | {
        |   switch_hold = INACTIVE;
        | }
  (default) -> NON_FUNCTIONAL
  NON_FUNCTIONAL -> EMEA_CONTROLS [order 1] : [CD_EMEA_CONTROLS_OPTION_A != NON_FUNCTIONAL...
      | || CD_EMEA_CONTROLS_OPTION_C != NON_FUNCTIONAL]
  NON_FUNCTIONAL -> SECOND_AUX_NA [order 2] : [CD_SECOND_AUX_FUNCTION != NON_FUNCTIONAL]
  data: din_SecondAuxSw input boolean; INACTIVE parameter uint8; ACTIVE parameter uint8; cmd_Diverter output boolean; CD_SECOND_AUX_SWITCH_DEBOUNCE parameter uint16; SINGLE_SHORT_BEEP parameter uint8; state_Buzzer data_store_memory double; state_Diverter input uint8; switch_hold local boolean; mode_LeftControl input uint8; MODE_LEFT_AUX2 parameter uint8; CD_EMEA_CONTROLS_OPTION_A parameter uint8; CD_EMEA_CONTROLS_OPTION_C parameter uint8; CD_SECOND_AUX_FUNCTION parameter uint8; NON_FUNCTIONAL parameter uint8

== stateflow chart MX_Gtwy_Control/Outputs/FuelPri_HVAC/Fuel_Primary_Relay/RelaySolenoid ==
  state INACTIVE
    en:dout_FuelPri = INACTIVE;
  note: Failure still allows operation of the machine, the internal feedback doesn't work
      | but we still have the relay feedback to guild us.
  state ACTIVE
    en:dout_FuelPri = ACTIVE;
    du:FuelTiming();
  state INACTIVE_OFF
    en:dout_FuelPri = INACTIVE;
  state FuelTiming [function]
    (default) -> (junction 3722)
    (junction 3722) -> (junction 3723) [order 1] : [(PullTime != UNLIMITED)...
        | && ((CD_FUEL_PRIMARY_ON_SECONDARY_FAILURE == NON_FUNCTIONAL) || (error_FuelSec == NORMAL))]
    (junction 3722) -> (junction 3724) [order 2]
    (junction 3723) -> (junction 3725) : {
        | outputTimer++;
        | }
    (junction 3725) -> (junction 3724)
  (default) -> INACTIVE
  INACTIVE_OFF -> INACTIVE : [(state_Engine == ENGINE_NOT_RUNNING)]
  ACTIVE -> INACTIVE [order 2] : [(state_Engine == ENGINE_NOT_RUNNING)]
  INACTIVE -> ACTIVE : [(CD_FUEL_PRIMARY_FUNCTION != NON_FUNCTIONAL)...
      | && (timer_Resolution == INACTIVE)...
      | && (state_Engine == ENGINE_CRANKING)...
      | && ((state_OutputError == NORMAL) || (state_OutputError == FAILURE))...
      | && (state_RelayError == NORMAL)]
      | {
      | outputTimer = 0;
      | }
  ACTIVE -> INACTIVE_OFF [order 1] : [(outputTimer >= PullTime)...
      | || !((state_OutputError == NORMAL) || (state_OutputError == FAILURE))...
      | || (state_RelayError != NORMAL)]
  data: dout_FuelPri output boolean; CD_FUEL_PRIMARY_FUNCTION parameter uint8; CD_FUEL_PRIMARY_ON_SECONDARY_FAILURE parameter uint8; state_OutputError input uint8; state_RelayError input uint8; outputTimer local uint16 =0; timer_Resolution input uint8 =0; error_FuelSec input double; state_Engine input uint8; UNLIMITED parameter uint16; ENGINE_NOT_RUNNING parameter uint8; ACTIVE parameter uint8; ENGINE_CRANKING parameter uint8; INACTIVE parameter uint8; NORMAL parameter uint8; PullTime input uint16; NON_FUNCTIONAL parameter uint8; FAILURE parameter uint8

== stateflow chart MX_Gtwy_Control/System/Derate/Derate ==
  state ECU
    state DERATE_LEVEL [parallel]
      settings: executionOrder=1
      note: %derate is the amount of torque/rpm allowed
          | i.e. 13% derate limits to 13% of available torque
          | and 13% of rpm range
          | No derate is 100%
      DERATE_LEVEL -> (junction 3781) : {
          | percent_Derate = 100;
          | }
      (junction 3781) -> (junction 3782)
      (junction 3782) -> (junction 3783) [order 1] : [(derate_Throttle < percent_Derate)]
      (junction 3783) -> (junction 3784) : {
          | percent_Derate = derate_Throttle;
          | }
      (junction 3782) -> (junction 3784) [order 2]
      (junction 3784) -> (junction 3785)
      (junction 3785) -> (junction 3786) [order 1] : [(CD_HP_MATCH_FUNCTION != NON_FUNCTIONAL)...
          | && (derate_HPMatch < percent_Derate)]
      (junction 3786) -> (junction 3787) : {
          | percent_Derate = derate_HPMatch;
          | }
      (junction 3785) -> (junction 3787) [order 2]
      (junction 3787) -> (junction 3788)
      (junction 3788) -> (junction 3789) [order 1] : [CD_DERATE_METHOD == 1]
      (junction 3789) -> (junction 3790) : {
          | torque_Derate = (torque_Indicated*uint16(percent_Derate))/uint16(100);
          | }
      (junction 3788) -> (junction 3790) [order 2] : {
          | torque_Derate = percent_Derate;
          | }
      (junction 3790) -> (junction 3791) : {
          | rpm_Derate = ecu_isocMinRPM + ((uint32(ecu_isocMaxRPM - ecu_isocMinRPM) * uint32(percent_Derate)) / uint16(100));
          | }
    state SEND_MSG [parallel]
      settings: executionOrder=2
      state NON_FUNCTIONAL
      state NORMAL
      state DERATE
        du:SendMsg_Derate_Now();
      state DISABLE_DERATE
        en:SendMsg_Derate_Now();
      (default) -> ECU.SEND_MSG.NON_FUNCTIONAL
      ECU.SEND_MSG.NON_FUNCTIONAL -> ECU.SEND_MSG.NORMAL : [(CD_ECU_FUNCTION != NON_FUNCTIONAL)]
      ECU.SEND_MSG.NORMAL -> ECU.SEND_MSG.DERATE : [(percent_Derate < 100)]
      DISABLE_DERATE -> ECU.SEND_MSG.NORMAL
      ECU.SEND_MSG.DERATE -> DISABLE_DERATE : [(percent_Derate == 100)]
    state RPM_FAULT [parallel]
      settings: executionOrder=3
      state UNRESPONSIVE
        state UNRESPONSIVE
          en: state_EngineSpeedDerate = UNRESPONSIVE;
        state DEBOUNCE
          en:debounceCount = 0;
          du:debounceCount++;
        (default) -> ECU.RPM_FAULT.UNRESPONSIVE.DEBOUNCE
        ECU.RPM_FAULT.UNRESPONSIVE.DEBOUNCE -> ECU.RPM_FAULT.UNRESPONSIVE.UNRESPONSIVE : [(debounceCount >= CD_DERATE_ENGINE_SPEED_DEBOUNCE_HIGH)]
      state NORMAL
        en:state_EngineSpeedDerate = NORMAL;
      state SHUTDOWN
        state SHUTDOWN
          en: state_EngineSpeedDerate = SHUTDOWN;
        state DEBOUNCE
          en:debounceCount = 0;
          du:debounceCount++;
        (default) -> ECU.RPM_FAULT.SHUTDOWN.DEBOUNCE
        ECU.RPM_FAULT.SHUTDOWN.DEBOUNCE -> ECU.RPM_FAULT.SHUTDOWN.SHUTDOWN : [(debounceCount >= CD_DERATE_ENGINE_SPEED_DEBOUNCE_SHUTDOWN)]
      (default) -> ECU.RPM_FAULT.NORMAL
      ECU.RPM_FAULT.NORMAL -> ECU.RPM_FAULT.UNRESPONSIVE : [(percent_Derate < 100)...
          | && (flt_EngineSpeed >= (rpm_Derate + CD_DERATE_ENGINE_SPEED_HIGH_OFFSET))]
      ECU.RPM_FAULT.UNRESPONSIVE -> ECU.RPM_FAULT.NORMAL [order 2] : [(percent_Derate ==100)...
          | || (flt_EngineSpeed < (rpm_Derate + CD_DERATE_ENGINE_SPEED_HIGH_OFFSET))]
      ECU.RPM_FAULT.UNRESPONSIVE -> ECU.RPM_FAULT.SHUTDOWN [order 1] : [(state_EngineSpeedDerate == UNRESPONSIVE)]
      ECU.RPM_FAULT.SHUTDOWN -> ECU.RPM_FAULT.NORMAL : [(state_EngineSpeedDerate != SHUTDOWN)...
          | && ((percent_Derate ==100)...
          |        || (flt_EngineSpeed < (rpm_Derate + CD_DERATE_ENGINE_SPEED_HIGH_OFFSET)))]
    state HP_MATCH [parallel]
      settings: executionOrder=4
      state NON_FUNCTIONAL
        en: derate_HPMatch = 100;
        en: state_HPMatch = NORMAL;
      state SEND_REQUEST
        en:
        num_Trys = 0;
        state SEND_MESSAGE
          en:
          SendMsg_SOFTRequest_Now();
        state WAIT_FOR_RESPONSE
          en:
          debounceRequest = 0;
          du:
          debounceRequest++;
        (default) -> SEND_MESSAGE
        SEND_MESSAGE -> WAIT_FOR_RESPONSE : [(state_CommECU == NORMAL)...
            | && (state_ECUHold == ACTIVE)]
        WAIT_FOR_RESPONSE -> SEND_MESSAGE : [(debounceRequest >= 180)]
            | {
            | num_Trys++;
            | }
      state REQUEST_RECEIVED
      state Cranking
      state DERATE
        en: derate_HPMatch = CD_MATCH_DERATE_PERCENT;
        en: state_HPMatch = FAILURE;
      (default) -> ECU.HP_MATCH.NON_FUNCTIONAL
      ECU.HP_MATCH.NON_FUNCTIONAL -> SEND_REQUEST : [(CD_HP_MATCH_FUNCTION != NON_FUNCTIONAL)]
      SEND_REQUEST -> REQUEST_RECEIVED [order 1] : [(ecu_HP != UNDEFINED)]
      REQUEST_RECEIVED -> ECU.HP_MATCH.DERATE : [(ecu_HP != CD_MACHINE_HP)]
      SEND_REQUEST -> Cranking [order 3] : [state_Engine == ENGINE_CRANKING]
      Cranking -> SEND_REQUEST : [state_Engine != ENGINE_CRANKING]
      SEND_REQUEST -> ECU.HP_MATCH.DERATE [order 2] : [(num_Trys > 3)]
  state NO_ECU
    en:
    state_HPMatch = NORMAL;
  (default) -> NO_ECU
  NO_ECU -> ECU : [(CD_ECU_FUNCTION != NON_FUNCTIONAL)]
  data: percent_Derate output int8; NON_FUNCTIONAL parameter uint8; CD_ECU_FUNCTION parameter uint8; rpm_Derate output uint16; state_EngineSpeedDerate output uint8; debounceCount local uint16; SHUTDOWN parameter uint8; NORMAL parameter uint8; CD_DERATE_ENGINE_SPEED_HIGH_OFFSET parameter uint16; CD_DERATE_ENGINE_SPEED_DEBOUNCE_HIGH parameter uint16; CD_DERATE_ENGINE_SPEED_DEBOUNCE_SHUTDOWN parameter uint16; flt_EngineSpeed input uint16; torque_Indicated input uint16; ecu_isocMinRPM input uint16; ecu_isocMaxRPM input uint16; UNRESPONSIVE parameter uint8; derate_Throttle input uint8; CD_HP_MATCH_FUNCTION parameter uint8; derate_HPMatch local uint8; state_HPMatch output uint8; num_Trys local uint8; state_CommECU input uint8; state_ECUHold input uint8; debounceRequest local uint16; ecu_HP input uint8; UNDEFINED parameter uint8; CD_MACHINE_HP parameter uint8; ACTIVE parameter uint8; CD_MATCH_DERATE_PERCENT parameter uint8; FAILURE parameter uint8; state_Engine input uint8; ENGINE_CRANKING parameter uint8; torque_Derate output uint16; CD_DERATE_METHOD parameter uint8

== stateflow chart MX_Gtwy_Control/Outputs/Starter/Starter ==
  state NON_FUNCTIONAL
    en:dout_Starter = INACTIVE;
  state MANUAL
    state ACTIVE
      en:dout_Starter = ACTIVE
    state INACTIVE
      en:dout_Starter = INACTIVE;
    (default) -> MANUAL.INACTIVE
    MANUAL.INACTIVE -> MANUAL.ACTIVE : [(state_Engine == ENGINE_CRANKING)...
        | && (state_VBattery != OORL)...
        | && (state_StarterOutput != ERROR_ON)...
        | && (state_StarterRelay != ERROR_ON)...
        | && (error_FuelSec == NORMAL)...
        | && (state_FuelPriOutput == NORMAL)...
        | && (state_FuelPriRelay == NORMAL)]
    MANUAL.ACTIVE -> MANUAL.INACTIVE : [(state_Engine != ENGINE_CRANKING)...
        | || (state_VBattery == OORL)...
        | || (error_FuelSec != NORMAL)...
        | || (state_FuelPriOutput != NORMAL)...
        | || (state_FuelPriRelay != NORMAL)]
  state AUTOMATED
    state ACTIVE
      en:dout_Starter = ACTIVE;
    state INACTIVE
      en:dout_Starter = INACTIVE;
    state DEBOUNCE_FOR_AUTO
      en:dout_Starter = ACTIVE;
      en:debounceAuto = 0;
      du:debounceAuto++;
    note: // ADD state to make sure that key is released before
        | allowing a it to crank again
    state AUTOMATED_START
      en:dout_Starter = ACTIVE;
      en:debounceAuto = 0;
      du:debounceAuto++;
    (default) -> AUTOMATED.INACTIVE
    AUTOMATED.INACTIVE -> AUTOMATED.ACTIVE : [(state_Engine == ENGINE_CRANKING)...
        | && (state_VBattery != OORL)...
        | && (state_StarterOutput != ERROR_ON)...
        | && (state_StarterRelay != ERROR_ON)...
        | && (error_FuelSec == NORMAL)...
        | && (state_FuelPriOutput == NORMAL)...
        | && (state_FuelPriRelay == NORMAL)]
    AUTOMATED.ACTIVE -> AUTOMATED.INACTIVE [order 1] : [(state_Engine != ENGINE_CRANKING)...
        | || (state_VBattery == OORL)...
        | || (error_FuelSec != NORMAL)...
        | || (state_FuelPriOutput != NORMAL)...
        | || (state_FuelPriRelay != NORMAL)]
    AUTOMATED.ACTIVE -> DEBOUNCE_FOR_AUTO [order 2] : [(flt_EngineSpeed >= level_StarterAutomatedEnagage)]
    AUTOMATED_START -> AUTOMATED.INACTIVE : [(flt_EngineSpeed >= level_StarterAutomatedDisengage)...
        | || (debounceAuto >= time_StarterAutomated)...
        | || (state_VBattery == OORL)...
        | || (error_FuelSec != NORMAL)...
        | || (state_FuelPriOutput != NORMAL)...
        | || (state_FuelPriRelay != NORMAL)]
    DEBOUNCE_FOR_AUTO -> AUTOMATED.INACTIVE [order 2] : [(state_Engine != ENGINE_CRANKING)...
        | || (state_VBattery == OORL)...
        | || (error_FuelSec != NORMAL)...
        | || (state_FuelPriOutput != NORMAL)...
        | || (state_FuelPriRelay != NORMAL)]
    DEBOUNCE_FOR_AUTO -> AUTOMATED_START [order 1] : [(debounceAuto >= CD_STARTER_AUTOMATED_DEBOUNCE_ACTIVATE)]
  (default) -> NON_FUNCTIONAL
  NON_FUNCTIONAL -> (junction 3911) : [(CD_STARTER_FUNCTION != NON_FUNCTIONAL)]
  (junction 3911) -> MANUAL [order 2]
  (junction 3911) -> AUTOMATED [order 1] : [(CD_STARTER_AUTOMATED_FUNCTION != NON_FUNCTIONAL)]
  data: state_Engine input uint8; state_VBattery input uint8; dout_Starter output boolean; CD_STARTER_FUNCTION parameter uint8; error_FuelSec input uint8; ACTIVE parameter uint8; ENGINE_CRANKING parameter uint8; INACTIVE parameter uint8; NORMAL parameter uint8; OORL parameter uint8; NON_FUNCTIONAL parameter uint8; state_StarterOutput input uint8; state_StarterRelay input uint8; ERROR_ON parameter uint8; state_FuelPriOutput input uint8; state_FuelPriRelay input uint8; level_StarterAutomatedEnagage input uint16; level_StarterAutomatedDisengage input uint16; time_StarterAutomated input uint16; flt_EngineSpeed input uint16; debounceAuto local uint16; CD_STARTER_AUTOMATED_FUNCTION parameter uint8; CD_STARTER_AUTOMATED_DEBOUNCE_ACTIVATE parameter uint16

== stateflow chart MX_Gtwy_Control/Outputs/Buzzer/Buzzer ==
  settings: updateMethod=DISCRETE  sampleTime=srate
  state No_Beep
    en:
    dout_Buzzer = INACTIVE;
    state_Buzzer = NO_BEEP;
    Beeps = 0;
  state Depth_Check
    state Warning_Zone
      state On
        en:
        dout_Buzzer = ACTIVE;
      state Off
        en:
        dout_Buzzer = INACTIVE;
      (default) -> Depth_Check.Warning_Zone.On
      Depth_Check.Warning_Zone.On -> Depth_Check.Warning_Zone.Off : [after(CD_WARNING_BEEP_LENGTH,tick)]
    state Below_Grade_Zone
      state On
        en:
        dout_Buzzer = ACTIVE;
      state Off
        en:
        dout_Buzzer = INACTIVE;
      (default) -> Depth_Check.Below_Grade_Zone.On
      Depth_Check.Below_Grade_Zone.Off -> Depth_Check.Below_Grade_Zone.On : [after(CD_CHIRP_OFF_LENGTH,tick)]
      Depth_Check.Below_Grade_Zone.On -> Depth_Check.Below_Grade_Zone.Off : [after(CD_CHIRP_ON_LENGTH,tick)]
    state Grade_Zone
      en:
      dout_Buzzer = ACTIVE;
      ex:
      dout_Buzzer = INACTIVE;
    (default) -> Warning_Zone
    Warning_Zone -> Grade_Zone [order 2] : [state_DepthBeep == DC_GRADE_BEEP]
    Grade_Zone -> Below_Grade_Zone [order 2] : [state_DepthBeep == DC_BELOW_GRADE_BEEP]
    Below_Grade_Zone -> Grade_Zone [order 2] : [state_DepthBeep == DC_GRADE_BEEP]
    Grade_Zone -> Warning_Zone [order 1] : [state_DepthBeep == DC_WARNING_BEEP]
    Warning_Zone -> Warning_Zone [order 1] : [after(delay_DepthBeep,tick)]
    Warning_Zone -> Below_Grade_Zone [order 3] : [state_DepthBeep == DC_BELOW_GRADE_BEEP]
    Below_Grade_Zone -> Warning_Zone [order 1] : [state_DepthBeep == DC_WARNING_BEEP]
  state Beep
    state On
      en:
      dout_Buzzer = ACTIVE;
    state Single_On
      en:
      dout_Buzzer = ACTIVE;
    state Single_Off
      en:
      dout_Buzzer = INACTIVE;
    state Multiple_On
      en:
      dout_Buzzer = ACTIVE;
    state Multiple_Off
      en:
      dout_Buzzer = INACTIVE;
    state Brief_Off
      en:
      dout_Buzzer = INACTIVE;
    state One_Beep_Medium
      en:
      dout_Buzzer = ACTIVE;
      on after(ONE_SECOND, tick):
      Beeps = 0;
    state One_Beep_Long
      en:
      dout_Buzzer = ACTIVE;
      on after(FIFTEEN_SECONDS, tick):
      Beeps = 0;
    (junction 3949) -> Beep.On [order 1] : [state_Buzzer == CONTINUOUS_BEEP]
        | {Beeps = 1;}
    (junction 3949) -> (junction 3950) [order 2]
    (junction 3950) -> Single_On [order 1] : [state_Buzzer == SINGLE_SHORT_BEEP]
        | {Beeps = 1;}
    (junction 3950) -> (junction 3951) [order 2]
    Single_On -> Single_Off [order 1] : [after(10,tick)]
        | {Beeps--;}
    Single_On -> Multiple_On [order 2] : [state_Buzzer == TRIPLE_SHORT_BEEP]
        | {Beeps = 3;}
    (junction 3951) -> Multiple_On [order 1] : [state_Buzzer == DOUBLE_SHORT_BEEP]
        | {Beeps = 2;}
    (junction 3951) -> (junction 3952) [order 2]
    (junction 3952) -> Multiple_On [order 1] : [state_Buzzer == TRIPLE_SHORT_BEEP]
        | {Beeps = 3;}
    (junction 3952) -> (junction 3953) [order 2]
    Brief_Off -> Multiple_On : [after(10,tick)]
    (junction 3953) -> Multiple_On [order 1] : [state_Buzzer == QUAD_SHORT_BEEP]
        | {Beeps = 4;}
    (junction 3953) -> (junction 3954) [order 2]
    Multiple_On -> Multiple_Off : [after(10,tick)]
        | {Beeps--;}
    Multiple_Off -> Multiple_On : [after(20,tick)]
    One_Beep_Medium -> Brief_Off : [state_Buzzer == TRIPLE_SHORT_BEEP...
        | && after(50, tick)]
        | {Beeps = 3;}
    (junction 3954) -> One_Beep_Medium [order 1] : [state_Buzzer == SINGLE_MEDIUM_BEEP]
        | {Beeps = 1;}
    (junction 3954) -> (junction 3955) [order 2]
    (junction 3955) -> One_Beep_Long [order 1] : [state_Buzzer == SINGLE_LONG_BEEP]
        | {Beeps = 1;}
  (default) -> No_Beep
  No_Beep -> Depth_Check [order 2] : [CD_DEPTH_CHECK_FUNCTION != NON_FUNCTIONAL...
      | && (state_DepthBeep & 0x80) == 0x80...
      | && state_DepthBeep != BEEP_NONE...
      | && state_System == SYS_NORMAL]
  Depth_Check -> No_Beep [order 1] : [(state_DepthBeep & 0x80) != 0x80...
      | || state_DepthBeep == BEEP_NONE...
      | || state_System != SYS_NORMAL]
  Depth_Check -> (junction 3949) [order 2] : [state_Buzzer != BEEP_NONE...
      | && state_Buzzer != NO_BEEP...
      | && state_System == SYS_NORMAL]
  No_Beep -> (junction 3949) [order 1] : [state_Buzzer != BEEP_NONE...
      | && state_Buzzer != NO_BEEP...
      | && state_System == SYS_NORMAL]
  Beep -> No_Beep : [state_Buzzer == NO_BEEP...
      | || !Beeps...
      | || state_System != SYS_NORMAL]
  (junction 3955) -> No_Beep [order 2]
  data: dout_Buzzer output boolean; Beeps local uint8; NO_BEEP parameter uint8; SINGLE_MEDIUM_BEEP parameter uint8; TRIPLE_SHORT_BEEP parameter uint8; DOUBLE_SHORT_BEEP parameter uint8; SINGLE_SHORT_BEEP parameter uint8; ONE_SECOND constant uint16 =99; CONTINUOUS_BEEP parameter uint8; ACTIVE parameter uint8; INACTIVE parameter uint8; state_Buzzer data_store_memory double; state_System input uint8; SYS_NORMAL parameter uint8; SINGLE_LONG_BEEP parameter uint8; FIFTEEN_SECONDS constant uint16 =1499; BEEP_NONE parameter uint8; QUAD_SHORT_BEEP parameter uint8; delay_DepthBeep input uint16; state_DepthBeep input uint8; DC_GRADE_BEEP parameter uint8; DC_WARNING_BEEP parameter uint8; DC_BELOW_GRADE_BEEP parameter uint8; CD_CHIRP_OFF_LENGTH parameter uint8; CD_CHIRP_ON_LENGTH parameter uint8; CD_DEPTH_CHECK_FUNCTION parameter uint8; CD_WARNING_BEEP_LENGTH parameter uint8; NON_FUNCTIONAL parameter uint8
